simulink model "MX_Gateway"  (Simulink 9.0)
settings: Solver=FixedStepDiscrete  StartTime=0.0  StopTime=100  FixedStep=0.01  RelTol=1e-3
values from neighbouring files (symbols this model uses):
  ACTIVE = Bobcat.Parameter; CreateDefine( ACTIVE                         ,1                          ,'Define' ,'defines.h' )    [defines.m]
  ACTIVE_MODE_ONE = Bobcat.Parameter; CreateDefine( ACTIVE_MODE_ONE                ,19                         ,'Define' ,'defines.h' )    [defines.m]
  AUTHENTICATED = Bobcat.Parameter; CreateDefine( AUTHENTICATED                  ,1                          ,'Define' ,'defines.h' )    [defines.m]
  AUX_EMEA_CONTROLS = Bobcat.Parameter; CreateDefine( AUX_EMEA_CONTROLS  ,1   ,'Define' ,'defines.h' )    [enums.m]
  AUX_FLOW_HIGH = Bobcat.Parameter; CreateDefine( AUX_FLOW_HIGH           ,4  ,'Define' ,'defines.h' )    [enums.m]
  AUX_FLOW_INACTIVE = Bobcat.Parameter; CreateDefine( AUX_FLOW_INACTIVE       ,0  ,'Define' ,'defines.h' )    [enums.m]
  AUX_FLOW_LOW = Bobcat.Parameter; CreateDefine( AUX_FLOW_LOW            ,1  ,'Define' ,'defines.h' )    [enums.m]
  AUX_FLOW_MEDIUM = Bobcat.Parameter; CreateDefine( AUX_FLOW_MEDIUM         ,2  ,'Define' ,'defines.h' )    [enums.m]
  AUX_FLOW_STANDARD = Bobcat.Parameter; CreateDefine( AUX_FLOW_STANDARD       ,3  ,'Define' ,'defines.h' )    [enums.m]
  AUX_NON_FUNCTIONAL = Bobcat.Parameter; CreateDefine( AUX_NON_FUNCTIONAL ,255 ,'Define' ,'defines.h' )    [enums.m]
  BASE_ACTIVE = Bobcat.Parameter; CreateDefine( BASE_ACTIVE                    ,253                        ,'Define' ,'defines.h' )    [defines.m]
  BASE_DETENT = Bobcat.Parameter; CreateDefine( BASE_DETENT                    ,1                          ,'Define' ,'defines.h' )    [defines.m]
  BEEP_NONE = Bobcat.Parameter; CreateDefine( BEEP_NONE                      ,255                        ,'Define' ,'defines.h' )    [defines.m]
  CD_AIR_FILTER_DEBOUNCE_NORMAL = Bobcat.Parameter; CreateAliasCD( CD_AIR_FILTER_DEBOUNCE_NORMAL                        ,uint16(500)                ,'ConstantDataPointer' ,'CONSTANT_DATA' ,'AIR_FILTER_DEBOUNCE_NORMAL')    [cdata.m]    (also in hc12_cdata.m; m4_cdata.m)
  CD_AIR_FILTER_DEBOUNCE_NOT_CONNECTED = Bobcat.Parameter; CreateAliasCD( CD_AIR_FILTER_DEBOUNCE_NOT_CONNECTED                 ,uint16(3000)               ,'ConstantDataPointer' ,'CONSTANT_DATA' ,'AIR_FILTER_DEBOUNCE_NOT_CONNECTED')    [cdata.m]    (also in hc12_cdata.m; m4_cdata.m)
  CD_AIR_FILTER_DEBOUNCE_PLUGGED = Bobcat.Parameter; CreateAliasCD( CD_AIR_FILTER_DEBOUNCE_PLUGGED                       ,uint16(3000)               ,'ConstantDataPointer' ,'CONSTANT_DATA' ,'AIR_FILTER_DEBOUNCE_PLUGGED')    [cdata.m]    (also in hc12_cdata.m; m4_cdata.m)
  CD_AIR_FILTER_FUNCTION = Bobcat.Parameter; CreateAliasCD( CD_AIR_FILTER_FUNCTION                               ,uint8(255)                 ,'ConstantDataPointer' ,'CONSTANT_DATA' ,'AIR_FILTER_FUNCTION')    [cdata.m]    (also in hc12_cdata.m; m4_cdata.m)
  CD_AIR_FILTER_PLUGGED_WEIGHT = Bobcat.Parameter; CreateAliasCD( CD_AIR_FILTER_PLUGGED_WEIGHT                         ,uint8(2)                   ,'ConstantDataPointer' ,'CONSTANT_DATA' ,'AIR_FILTER_PLUGGED_WEIGHT')    [cdata.m]    (also in hc12_cdata.m; m4_cdata.m)
  CD_AIR_FILTER_UNPLUGGED_WEIGHT = Bobcat.Parameter; CreateAliasCD( CD_AIR_FILTER_UNPLUGGED_WEIGHT                       ,uint8(1)                   ,'ConstantDataPointer' ,'CONSTANT_DATA' ,'AIR_FILTER_UNPLUGGED_WEIGHT')    [cdata.m]    (also in hc12_cdata.m; m4_cdata.m)
  CD_AUTOIDLE_ARMBAR_DELAY = Bobcat.Parameter; CreateAliasCD( CD_AUTOIDLE_ARMBAR_DELAY                             ,uint8(2)                   ,'ConstantDataPointer' ,'CONSTANT_DATA' ,'AUTOIDLE_ARMBAR_DELAY')    [cdata.m]    (also in hc12_cdata.m; m4_cdata.m)
  CD_AUTOIDLE_FUNCTION = Bobcat.Parameter; CreateAliasCD( CD_AUTOIDLE_FUNCTION                                 ,uint8(255)                 ,'ConstantDataPointer' ,'CONSTANT_DATA' ,'AUTOIDLE_FUNCTION')    [cdata.m]    (also in hc12_cdata.m; m4_cdata.m)
  CD_AUTOIDLE_IDLE_DELAY = Bobcat.Parameter; CreateAliasCD( CD_AUTOIDLE_IDLE_DELAY                               ,uint8(40)                  ,'ConstantDataPointer' ,'CONSTANT_DATA' ,'AUTOIDLE_IDLE_DELAY')    [cdata.m]    (also in hc12_cdata.m; m4_cdata.m)
  CD_AUTOIDLE_LOW_IDLE_START = Bobcat.Parameter; CreateAliasCD( CD_AUTOIDLE_LOW_IDLE_START                           ,uint8(255)                 ,'ConstantDataPointer' ,'CONSTANT_DATA' ,'AUTOIDLE_LOW_IDLE_START')    [cdata.m]    (also in hc12_cdata.m; m4_cdata.m)
  CD_AUTOIDLE_PRESSURE_OFF = Bobcat.Parameter; CreateAliasCD( CD_AUTOIDLE_PRESSURE_OFF                             ,uint16(300)                ,'ConstantDataPointer' ,'CONSTANT_DATA' ,'AUTOIDLE_PRESSURE_OFF')    [cdata.m]    (also in hc12_cdata.m; m4_cdata.m)
  CD_AUTOIDLE_PRESSURE_ON = Bobcat.Parameter; CreateAliasCD( CD_AUTOIDLE_PRESSURE_ON                              ,uint16(270)                ,'ConstantDataPointer' ,'CONSTANT_DATA' ,'AUTOIDLE_PRESSURE_ON')    [cdata.m]    (also in hc12_cdata.m; m4_cdata.m)
  CD_AUTOSHIFT_FUNCTION = Bobcat.Parameter; CreateAliasCD( CD_AUTOSHIFT_FUNCTION                                ,uint8(255)                 ,'ConstantDataPointer' ,'CONSTANT_DATA' ,'AUTOSHIFT_FUNCTION')    [cdata.m]    (also in hc12_cdata.m; m4_cdata.m)
  CD_AUTOSHIFT_PHIGH1 = Bobcat.Parameter; CreateAliasCD( CD_AUTOSHIFT_PHIGH1                                  ,uint16(205)                ,'ConstantDataPointer' ,'CONSTANT_DATA' ,'AUTOSHIFT_PHIGH1')    [cdata.m]    (also in hc12_cdata.m; m4_cdata.m)
  CD_AUTOSHIFT_PHIGH2 = Bobcat.Parameter; CreateAliasCD( CD_AUTOSHIFT_PHIGH2                                  ,uint16(193)                ,'ConstantDataPointer' ,'CONSTANT_DATA' ,'AUTOSHIFT_PHIGH2')    [cdata.m]    (also in hc12_cdata.m; m4_cdata.m)
  CD_AUTOSHIFT_PHIGH_TEMP1 = Bobcat.Parameter; CreateAliasCD( CD_AUTOSHIFT_PHIGH_TEMP1                             ,int16(70)                  ,'ConstantDataPointer' ,'CONSTANT_DATA' ,'AUTOSHIFT_PHIGH_TEMP1')    [cdata.m]    (also in hc12_cdata.m; m4_cdata.m)
  CD_AUTOSHIFT_PHIGH_TEMP2 = Bobcat.Parameter; CreateAliasCD( CD_AUTOSHIFT_PHIGH_TEMP2                             ,int16(160)                 ,'ConstantDataPointer' ,'CONSTANT_DATA' ,'AUTOSHIFT_PHIGH_TEMP2')    [cdata.m]    (also in hc12_cdata.m; m4_cdata.m)
  CD_AUTOSHIFT_PLOW1 = Bobcat.Parameter; CreateAliasCD( CD_AUTOSHIFT_PLOW1                                   ,uint16(130)                ,'ConstantDataPointer' ,'CONSTANT_DATA' ,'AUTOSHIFT_PLOW1')    [cdata.m]    (also in hc12_cdata.m; m4_cdata.m)
  CD_AUTOSHIFT_PLOW2 = Bobcat.Parameter; CreateAliasCD( CD_AUTOSHIFT_PLOW2                                   ,uint16(100)                ,'ConstantDataPointer' ,'CONSTANT_DATA' ,'AUTOSHIFT_PLOW2')    [cdata.m]    (also in hc12_cdata.m; m4_cdata.m)
  CD_AUTOSHIFT_PLOW_TEMP1 = Bobcat.Parameter; CreateAliasCD( CD_AUTOSHIFT_PLOW_TEMP1                              ,int16(20)                  ,'ConstantDataPointer' ,'CONSTANT_DATA' ,'AUTOSHIFT_PLOW_TEMP1')    [cdata.m]    (also in hc12_cdata.m; m4_cdata.m)
  CD_AUTOSHIFT_PLOW_TEMP2 = Bobcat.Parameter; CreateAliasCD( CD_AUTOSHIFT_PLOW_TEMP2                              ,int16(80)                  ,'ConstantDataPointer' ,'CONSTANT_DATA' ,'AUTOSHIFT_PLOW_TEMP2')    [cdata.m]    (also in hc12_cdata.m; m4_cdata.m)
  CD_AUTOSHIFT_TRAVEL_DELAY = Bobcat.Parameter; CreateAliasCD( CD_AUTOSHIFT_TRAVEL_DELAY                            ,uint8(32)                  ,'ConstantDataPointer' ,'CONSTANT_DATA' ,'AUTOSHIFT_TRAVEL_DELAY')    [cdata.m]    (also in hc12_cdata.m; m4_cdata.m)
  CD_AUX2_CRACKPOINT_CURRENT = Bobcat.Parameter; CreateAliasCD( CD_AUX2_CRACKPOINT_CURRENT                           ,fi(0,0,16,12,'hex','0c28') ,'ConstantDataPointer' ,'CONSTANT_DATA' ,'AUX2_CRACKPOINT_CURRENT')    [cdata.m]    (also in hc12_cdata.m; m4_cdata.m)
  CD_AUX2_FLOW_LOW_FUNCTION = Bobcat.Parameter; CreateAliasCD( CD_AUX2_FLOW_LOW_FUNCTION                            ,uint8(255)                 ,'ConstantDataPointer' ,'CONSTANT_DATA' ,'AUX2_FLOW_LOW_FUNCTION')    [cdata.m]    (also in hc12_cdata.m; m4_cdata.m)
  CD_AUX2_FLOW_MED_FUNCTION = Bobcat.Parameter; CreateAliasCD( CD_AUX2_FLOW_MED_FUNCTION                            ,uint8(255)                 ,'ConstantDataPointer' ,'CONSTANT_DATA' ,'AUX2_FLOW_MED_FUNCTION')    [cdata.m]    (also in hc12_cdata.m; m4_cdata.m)
  CD_AUX2_HI_FLOW_PERCENT = Bobcat.Parameter; CreateAliasCD( CD_AUX2_HI_FLOW_PERCENT                              ,fi(0,0,16,16,'hex','ffff') ,'ConstantDataPointer' ,'CONSTANT_DATA' ,'AUX2_HI_FLOW_PERCENT')    [cdata.m]    (also in hc12_cdata.m; m4_cdata.m)
  CD_AUX2_LOW_FLOW_PERCENT = Bobcat.Parameter; CreateAliasCD( CD_AUX2_LOW_FLOW_PERCENT                             ,fi(0,0,16,16,'hex','7333') ,'ConstantDataPointer' ,'CONSTANT_DATA' ,'AUX2_LOW_FLOW_PERCENT')    [cdata.m]    (also in hc12_cdata.m; m4_cdata.m)
  CD_AUX2_MAX_FLOW_CURRENT = Bobcat.Parameter; CreateAliasCD( CD_AUX2_MAX_FLOW_CURRENT                             ,fi(0,0,16,12,'hex','14cc') ,'ConstantDataPointer' ,'CONSTANT_DATA' ,'AUX2_MAX_FLOW_CURRENT')    [cdata.m]    (also in hc12_cdata.m; m4_cdata.m)
  CD_AUX2_MED_FLOW_PERCENT = Bobcat.Parameter; CreateAliasCD( CD_AUX2_MED_FLOW_PERCENT                             ,fi(0,0,16,16,'hex','9999') ,'ConstantDataPointer' ,'CONSTANT_DATA' ,'AUX2_MED_FLOW_PERCENT')    [cdata.m]    (also in hc12_cdata.m; m4_cdata.m)
  CD_AUX_CRACKPOINT_CURRENT = Bobcat.Parameter; CreateAliasCD( CD_AUX_CRACKPOINT_CURRENT                            ,fi(0,0,16,12,'hex','0000') ,'ConstantDataPointer' ,'CONSTANT_DATA' ,'AUX_CRACKPOINT_CURRENT')    [cdata.m]    (also in hc12_cdata.m; m4_cdata.m)
  CD_AUX_DETENT_FLASH_TIME = Bobcat.Parameter; CreateAliasCD( CD_AUX_DETENT_FLASH_TIME                             ,uint16(72)                 ,'ConstantDataPointer' ,'CONSTANT_DATA' ,'AUX_DETENT_FLASH_TIME')    [cdata.m]    (also in hc12_cdata.m; m4_cdata.m)
  CD_AUX_DETENT_HOLD_TIME = Bobcat.Parameter; CreateAliasCD( CD_AUX_DETENT_HOLD_TIME                              ,uint16(100)                ,'ConstantDataPointer' ,'CONSTANT_DATA' ,'AUX_DETENT_HOLD_TIME')    [cdata.m]    (also in hc12_cdata.m; m4_cdata.m)
  CD_AUX_FULL_ON_AUX1 = Bobcat.Parameter; CreateAliasCD( CD_AUX_FULL_ON_AUX1                                  ,fi(0,0,16,12,'hex','10cd') ,'ConstantDataPointer' ,'CONSTANT_DATA' ,'AUX_FULL_ON_AUX1')    [cdata.m]    (also in hc12_cdata.m; m4_cdata.m)
  CD_AUX_FULL_ON_AUX2 = Bobcat.Parameter; CreateAliasCD( CD_AUX_FULL_ON_AUX2                                  ,fi(0,0,16,12,'hex','139d') ,'ConstantDataPointer' ,'CONSTANT_DATA' ,'AUX_FULL_ON_AUX2')    [cdata.m]    (also in hc12_cdata.m; m4_cdata.m)
  CD_AUX_FULL_ON_AUX3 = Bobcat.Parameter; CreateAliasCD( CD_AUX_FULL_ON_AUX3                                  ,fi(0,0,16,12,'hex','17d7') ,'ConstantDataPointer' ,'CONSTANT_DATA' ,'AUX_FULL_ON_AUX3')    [cdata.m]    (also in hc12_cdata.m; m4_cdata.m)
  CD_AUX_FUNCTION = Bobcat.Parameter; CreateAliasCD( CD_AUX_FUNCTION                                      ,uint8(0)                   ,'ConstantDataPointer' ,'CONSTANT_DATA' ,'AUX_FUNCTION')    [cdata.m]    (also in hc12_cdata.m; m4_cdata.m)
  CD_AUX_RATE_LIMIT_CRACKPOINT = Bobcat.Parameter; CreateAliasCD( CD_AUX_RATE_LIMIT_CRACKPOINT                         ,fi(0,0,16,12,'hex','0800') ,'ConstantDataPointer' ,'CONSTANT_DATA' ,'AUX_RATE_LIMIT_CRACKPOINT')    [cdata.m]    (also in hc12_cdata.m; m4_cdata.m)
  CD_AUX_RATE_LIMIT_CRACKPOINT_RATE = Bobcat.Parameter; CreateAliasCD( CD_AUX_RATE_LIMIT_CRACKPOINT_RATE                    ,fi(0,1,32,12,'int',102400) ,'ConstantDataPointer' ,'CONSTANT_DATA' ,'AUX_RATE_LIMIT_CRACKPOINT_RATE')    [cdata.m]    (also in hc12_cdata.m; m4_cdata.m)
  CD_AUX_RATE_LIMIT_FUNCTION = Bobcat.Parameter; CreateAliasCD( CD_AUX_RATE_LIMIT_FUNCTION                           ,uint8(255)                 ,'ConstantDataPointer' ,'CONSTANT_DATA' ,'AUX_RATE_LIMIT_FUNCTION')    [cdata.m]    (also in hc12_cdata.m; m4_cdata.m)
  CD_AUX_RATE_LIMIT_MAX_ENG_SPD = Bobcat.Parameter; CreateAliasCD( CD_AUX_RATE_LIMIT_MAX_ENG_SPD                        ,uint16(1600)               ,'ConstantDataPointer' ,'CONSTANT_DATA' ,'AUX_RATE_LIMIT_MAX_ENG_SPD')    [cdata.m]    (also in hc12_cdata.m; m4_cdata.m)
  CD_AUX_RATE_LIMIT_MIN_ENG_SPD = Bobcat.Parameter; CreateAliasCD( CD_AUX_RATE_LIMIT_MIN_ENG_SPD                        ,uint16(100)                ,'ConstantDataPointer' ,'CONSTANT_DATA' ,'AUX_RATE_LIMIT_MIN_ENG_SPD')    [cdata.m]    (also in hc12_cdata.m; m4_cdata.m)
  CD_AUX_RATE_LIMIT_NORMAL_RATE = Bobcat.Parameter; CreateAliasCD( CD_AUX_RATE_LIMIT_NORMAL_RATE                        ,fi(0,1,32,12,'int',2048)   ,'ConstantDataPointer' ,'CONSTANT_DATA' ,'AUX_RATE_LIMIT_NORMAL_RATE')    [cdata.m]    (also in hc12_cdata.m; m4_cdata.m)
  CD_AUXPOT_CAL_DEADBAND_HIGH = Bobcat.Parameter; CreateAliasCD( CD_AUXPOT_CAL_DEADBAND_HIGH                          ,uint16(574)                ,'ConstantDataPointer' ,'CONSTANT_DATA' ,'AUXPOT_CAL_DEADBAND_HIGH')    [cdata.m]    (also in hc12_cdata.m; m4_cdata.m)
  CD_AUXPOT_CAL_DEADBAND_LOW = Bobcat.Parameter; CreateAliasCD( CD_AUXPOT_CAL_DEADBAND_LOW                           ,uint16(424)                ,'ConstantDataPointer' ,'CONSTANT_DATA' ,'AUXPOT_CAL_DEADBAND_LOW')    [cdata.m]    (also in hc12_cdata.m; m4_cdata.m)
  CD_AUXPOT_CENTER_RATIO = Bobcat.Parameter; CreateAliasCD( CD_AUXPOT_CENTER_RATIO                               ,uint16(500)                ,'ConstantDataPointer' ,'CONSTANT_DATA' ,'AUXPOT_CENTER_RATIO')    [cdata.m]    (also in hc12_cdata.m; m4_cdata.m)
  CD_AUXPOT_DEADBAND = Bobcat.Parameter; CreateAliasCD( CD_AUXPOT_DEADBAND                                   ,uint8(38)                  ,'ConstantDataPointer' ,'CONSTANT_DATA' ,'AUXPOT_DEADBAND')    [cdata.m]    (also in hc12_cdata.m; m4_cdata.m)
  CD_AUXPOT_FUNCTION = Bobcat.Parameter; CreateAliasCD( CD_AUXPOT_FUNCTION                                   ,uint8(0)                   ,'ConstantDataPointer' ,'CONSTANT_DATA' ,'AUXPOT_FUNCTION')    [cdata.m]    (also in hc12_cdata.m; m4_cdata.m)
  CD_AUXPOT_RATIO_GAIN = Bobcat.Parameter; CreateAliasCD( CD_AUXPOT_RATIO_GAIN                                 ,uint16(1000)               ,'ConstantDataPointer' ,'CONSTANT_DATA' ,'AUXPOT_RATIO_GAIN')    [cdata.m]    (also in hc12_cdata.m; m4_cdata.m)
  CD_CAN_THROTTLE_FUNCTION = Bobcat.Parameter; CreateAliasCD( CD_CAN_THROTTLE_FUNCTION                             ,uint8(255)                 ,'ConstantDataPointer' ,'CONSTANT_DATA' ,'CAN_THROTTLE_FUNCTION')    [cdata.m]    (also in hc12_cdata.m; m4_cdata.m)
  CD_CAN_THROTTLE_REVERSE = Bobcat.Parameter; CreateAliasCD( CD_CAN_THROTTLE_REVERSE                              ,uint8(255)                 ,'ConstantDataPointer' ,'CONSTANT_DATA' ,'CAN_THROTTLE_REVERSE')    [cdata.m]    (also in hc12_cdata.m; m4_cdata.m)
  CD_CONSOLE_DEBOUNCE_ABSENT = Bobcat.Parameter; CreateAliasCD( CD_CONSOLE_DEBOUNCE_ABSENT                           ,uint16(12)                 ,'ConstantDataPointer' ,'CONSTANT_DATA' ,'CONSOLE_DEBOUNCE_ABSENT')    [cdata.m]    (also in hc12_cdata.m; m4_cdata.m)
  CD_CONSOLE_DEBOUNCE_OORH = Bobcat.Parameter; CreateAliasCD( CD_CONSOLE_DEBOUNCE_OORH                             ,uint16(12)                 ,'ConstantDataPointer' ,'CONSTANT_DATA' ,'CONSOLE_DEBOUNCE_OORH')    [cdata.m]    (also in hc12_cdata.m; m4_cdata.m)
  CD_CONSOLE_DEBOUNCE_OORL = Bobcat.Parameter; CreateAliasCD( CD_CONSOLE_DEBOUNCE_OORL                             ,uint16(12)                 ,'ConstantDataPointer' ,'CONSTANT_DATA' ,'CONSOLE_DEBOUNCE_OORL')    [cdata.m]    (also in hc12_cdata.m; m4_cdata.m)
  CD_CONSOLE_DEBOUNCE_PRESENT = Bobcat.Parameter; CreateAliasCD( CD_CONSOLE_DEBOUNCE_PRESENT                          ,uint16(12)                 ,'ConstantDataPointer' ,'CONSTANT_DATA' ,'CONSOLE_DEBOUNCE_PRESENT')    [cdata.m]    (also in hc12_cdata.m; m4_cdata.m)
  CD_CONSOLE_FUNCTION = Bobcat.Parameter; CreateAliasCD( CD_CONSOLE_FUNCTION                                  ,uint8(0)                   ,'ConstantDataPointer' ,'CONSTANT_DATA' ,'CONSOLE_FUNCTION')    [cdata.m]    (also in hc12_cdata.m; m4_cdata.m)
  CD_CONSOLE_OORH = Bobcat.Parameter; CreateAliasCD( CD_CONSOLE_OORH                                      ,uint16(740)                ,'ConstantDataPointer' ,'CONSTANT_DATA' ,'CONSOLE_OORH')    [cdata.m]    (also in hc12_cdata.m; m4_cdata.m)
  CD_CONSOLE_OORL = Bobcat.Parameter; CreateAliasCD( CD_CONSOLE_OORL                                      ,uint16(10)                 ,'ConstantDataPointer' ,'CONSTANT_DATA' ,'CONSOLE_OORL')    [cdata.m]    (also in hc12_cdata.m; m4_cdata.m)
  CD_CONSOLE_PRESENT_HIGH = Bobcat.Parameter; CreateAliasCD( CD_CONSOLE_PRESENT_HIGH                              ,uint16(515)                ,'ConstantDataPointer' ,'CONSTANT_DATA' ,'CONSOLE_PRESENT_HIGH')    [cdata.m]    (also in hc12_cdata.m; m4_cdata.m)
  CD_CONSOLE_PRESENT_LOW = Bobcat.Parameter; CreateAliasCD( CD_CONSOLE_PRESENT_LOW                               ,uint16(375)                ,'ConstantDataPointer' ,'CONSTANT_DATA' ,'CONSOLE_PRESENT_LOW')    [cdata.m]    (also in hc12_cdata.m; m4_cdata.m)
  CD_DELUXE_GEN5_5_FUNCTION = Bobcat.Parameter; CreateAliasCD( CD_DELUXE_GEN5_5_FUNCTION                            ,uint8(255)                 ,'ConstantDataPointer' ,'CONSTANT_DATA' ,'DELUXE_GEN5_5_FUNCTION')    [cdata.m]    (also in hc12_cdata.m; m4_cdata.m)
  CD_DELUXE_GEN5_FUNCTION = Bobcat.Parameter; CreateAliasCD( CD_DELUXE_GEN5_FUNCTION                              ,uint8(255)                 ,'ConstantDataPointer' ,'CONSTANT_DATA' ,'DELUXE_GEN5_FUNCTION')    [cdata.m]    (also in hc12_cdata.m; m4_cdata.m)
  CD_DERATE_ENGINE_SPEED_DEBOUNCE_HIGH = Bobcat.Parameter; CreateAliasCD( CD_DERATE_ENGINE_SPEED_DEBOUNCE_HIGH                 ,uint16(200)                ,'ConstantDataPointer' ,'CONSTANT_DATA' ,'DERATE_ENGINE_SPEED_DEBOUNCE_HIGH')    [cdata.m]    (also in hc12_cdata.m; m4_cdata.m)
  CD_DERATE_ENGINE_SPEED_DEBOUNCE_SHUTDOWN = Bobcat.Parameter; CreateAliasCD( CD_DERATE_ENGINE_SPEED_DEBOUNCE_SHUTDOWN             ,uint16(1000)               ,'ConstantDataPointer' ,'CONSTANT_DATA' ,'DERATE_ENGINE_SPEED_DEBOUNCE_SHUTDOWN')    [cdata.m]    (also in hc12_cdata.m; m4_cdata.m)
  CD_DERATE_ENGINE_SPEED_HIGH_OFFSET = Bobcat.Parameter; CreateAliasCD( CD_DERATE_ENGINE_SPEED_HIGH_OFFSET                   ,uint16(200)                ,'ConstantDataPointer' ,'CONSTANT_DATA' ,'DERATE_ENGINE_SPEED_HIGH_OFFSET')    [cdata.m]    (also in hc12_cdata.m; m4_cdata.m)
  CD_DERATE_METHOD = Bobcat.Parameter; CreateAliasCD( CD_DERATE_METHOD                                     ,uint8(0)                   ,'ConstantDataPointer' ,'CONSTANT_DATA' ,'DERATE_METHOD')    [cdata.m]    (also in hc12_cdata.m; m4_cdata.m)
  CD_DUMP_VALVE_FUNCTION = Bobcat.Parameter; CreateAliasCD( CD_DUMP_VALVE_FUNCTION                               ,uint8(255)                 ,'ConstantDataPointer' ,'CONSTANT_DATA' ,'DUMP_VALVE_FUNCTION')    [cdata.m]    (also in hc12_cdata.m; m4_cdata.m)
  CD_DUMP_VALVE_ON_CURRENT = Bobcat.Parameter; CreateAliasCD( CD_DUMP_VALVE_ON_CURRENT                             ,fi(0,0,16,12,'hex','ffff') ,'ConstantDataPointer' ,'CONSTANT_DATA' ,'DUMP_VALVE_ON_CURRENT')    [cdata.m]    (also in hc12_cdata.m; m4_cdata.m)
  CD_ECO_MODE_THROTTLE_REDUCTION = Bobcat.Parameter; CreateAliasCD( CD_ECO_MODE_THROTTLE_REDUCTION                       ,uint16(850)                ,'ConstantDataPointer' ,'CONSTANT_DATA' ,'ECO_MODE_THROTTLE_REDUCTION')    [cdata.m]    (also in hc12_cdata.m; m4_cdata.m)
  CD_ECT_FILTER_GAIN = Bobcat.Parameter; CreateAliasCD( CD_ECT_FILTER_GAIN                                   ,uint8(6)                   ,'ConstantDataPointer' ,'CONSTANT_DATA' ,'ECT_FILTER_GAIN')    [cdata.m]    (also in hc12_cdata.m; m4_cdata.m)
  CD_ECU_FUNCTION = Bobcat.Parameter; CreateAliasCD( CD_ECU_FUNCTION                                      ,uint8(255)                 ,'ConstantDataPointer' ,'CONSTANT_DATA' ,'ECU_FUNCTION')    [cdata.m]    (also in hc12_cdata.m; m4_cdata.m)
  CD_ECU_HOLD_DEBOUNCE_ERROR_ON = Bobcat.Parameter; CreateAliasCD( CD_ECU_HOLD_DEBOUNCE_ERROR_ON                        ,uint16(20)                 ,'ConstantDataPointer' ,'CONSTANT_DATA' ,'ECU_HOLD_DEBOUNCE_ERROR_ON')    [cdata.m]    (also in hc12_cdata.m; m4_cdata.m)
  CD_EMEA_CONTROLS_HOLD_TIME = Bobcat.Parameter; CreateAliasCD( CD_EMEA_CONTROLS_HOLD_TIME                           ,uint16(30)                 ,'ConstantDataPointer' ,'CONSTANT_DATA' ,'EMEA_CONTROLS_HOLD_TIME')    [cdata.m]    (also in hc12_cdata.m; m4_cdata.m)
  CD_EMEA_CONTROLS_OPTION_A = Bobcat.Parameter; CreateAliasCD( CD_EMEA_CONTROLS_OPTION_A                            ,uint8(255)                 ,'ConstantDataPointer' ,'CONSTANT_DATA' ,'EMEA_CONTROLS_OPTION_A')    [cdata.m]    (also in hc12_cdata.m; m4_cdata.m)
  CD_EMEA_CONTROLS_OPTION_C = Bobcat.Parameter; CreateAliasCD( CD_EMEA_CONTROLS_OPTION_C                            ,uint8(255)                 ,'ConstantDataPointer' ,'CONSTANT_DATA' ,'EMEA_CONTROLS_OPTION_C')    [cdata.m]    (also in hc12_cdata.m; m4_cdata.m)
  CD_EMEA_CONTROLS_REVERSE = Bobcat.Parameter; CreateAliasCD( CD_EMEA_CONTROLS_REVERSE                             ,uint8(255)                 ,'ConstantDataPointer' ,'CONSTANT_DATA' ,'EMEA_CONTROLS_REVERSE')    [cdata.m]    (also in hc12_cdata.m; m4_cdata.m)
  CD_EMEA_ELECTRIC_VERSION = Bobcat.Parameter; CreateAliasCD( CD_EMEA_ELECTRIC_VERSION                             ,uint8(0)                   ,'ConstantDataPointer' ,'CONSTANT_DATA' ,'EMEA_ELECTRIC_VERSION')    [cdata.m]    (also in hc12_cdata.m; m4_cdata.m)
  CD_EMEA_POT_CAL_DEADBAND_HIGH = Bobcat.Parameter; CreateAliasCD( CD_EMEA_POT_CAL_DEADBAND_HIGH                        ,uint16(574)                ,'ConstantDataPointer' ,'CONSTANT_DATA' ,'EMEA_POT_CAL_DEADBAND_HIGH')    [cdata.m]    (also in hc12_cdata.m; m4_cdata.m)
  CD_EMEA_POT_CAL_DEADBAND_LOW = Bobcat.Parameter; CreateAliasCD( CD_EMEA_POT_CAL_DEADBAND_LOW                         ,uint16(424)                ,'ConstantDataPointer' ,'CONSTANT_DATA' ,'EMEA_POT_CAL_DEADBAND_LOW')    [cdata.m]    (also in hc12_cdata.m; m4_cdata.m)
  CD_ENGINE_RPM_FACTOR = Bobcat.Parameter; CreateAliasCD( CD_ENGINE_RPM_FACTOR                                 ,uint8(35)                  ,'ConstantDataPointer' ,'CONSTANT_DATA' ,'ENGINE_RPM_FACTOR')    [cdata.m]    (also in hc12_cdata.m; m4_cdata.m)
  CD_ENGINE_SPEED_FILTER_FAST_VALUE = Bobcat.Parameter; CreateAliasCD( CD_ENGINE_SPEED_FILTER_FAST_VALUE                    ,uint8(1)                   ,'ConstantDataPointer' ,'CONSTANT_DATA' ,'ENGINE_SPEED_FILTER_FAST_VALUE')    [cdata.m]    (also in hc12_cdata.m; m4_cdata.m)
  CD_ENGINE_SPEED_FILTER_SLOW_VALUE = Bobcat.Parameter; CreateAliasCD( CD_ENGINE_SPEED_FILTER_SLOW_VALUE                    ,uint8(4)                   ,'ConstantDataPointer' ,'CONSTANT_DATA' ,'ENGINE_SPEED_FILTER_SLOW_VALUE')    [cdata.m]    (also in hc12_cdata.m; m4_cdata.m)
  CD_ENGINE_SPEED_RUNNING = Bobcat.Parameter; CreateAliasCD( CD_ENGINE_SPEED_RUNNING                              ,uint16(800)                ,'ConstantDataPointer' ,'CONSTANT_DATA' ,'ENGINE_SPEED_RUNNING')    [cdata.m]    (also in hc12_cdata.m; m4_cdata.m)
  CD_ENGINE_START_DELAY_DEBOUNCE_RPM_NO_SIGNAL = Bobcat.Parameter; CreateAliasCD( CD_ENGINE_START_DELAY_DEBOUNCE_RPM_NO_SIGNAL         ,uint16(0)                  ,'ConstantDataPointer' ,'CONSTANT_DATA' ,'ENGINE_START_DELAY_DEBOUNCE_RPM_NO_SIGNAL')    [cdata.m]    (also in hc12_cdata.m; m4_cdata.m)
  CD_ENGINE_START_DELAY_OFF_TIME = Bobcat.Parameter; CreateAliasCD( CD_ENGINE_START_DELAY_OFF_TIME                       ,uint16(0)                  ,'ConstantDataPointer' ,'CONSTANT_DATA' ,'ENGINE_START_DELAY_OFF_TIME')    [cdata.m]    (also in hc12_cdata.m; m4_cdata.m)
  CD_ENGINE_START_DELAY_RPM_NO_SIGNAL = Bobcat.Parameter; CreateAliasCD( CD_ENGINE_START_DELAY_RPM_NO_SIGNAL                  ,uint16(0)                  ,'ConstantDataPointer' ,'CONSTANT_DATA' ,'ENGINE_START_DELAY_RPM_NO_SIGNAL')    [cdata.m]    (also in hc12_cdata.m; m4_cdata.m)
  CD_EOP_DEBOUNCE_EXTREMELY_LOW = Bobcat.Parameter; CreateAliasCD( CD_EOP_DEBOUNCE_EXTREMELY_LOW                        ,uint16(50)                 ,'ConstantDataPointer' ,'CONSTANT_DATA' ,'EOP_DEBOUNCE_EXTREMELY_LOW')    [cdata.m]    (also in hc12_cdata.m; m4_cdata.m)
  CD_EOP_DEBOUNCE_NORMAL = Bobcat.Parameter; CreateAliasCD( CD_EOP_DEBOUNCE_NORMAL                               ,uint16(50)                 ,'ConstantDataPointer' ,'CONSTANT_DATA' ,'EOP_DEBOUNCE_NORMAL')    [cdata.m]    (also in hc12_cdata.m; m4_cdata.m)
  CD_EOP_DEBOUNCE_SHUTDOWN = Bobcat.Parameter; CreateAliasCD( CD_EOP_DEBOUNCE_SHUTDOWN                             ,uint16(100)                ,'ConstantDataPointer' ,'CONSTANT_DATA' ,'EOP_DEBOUNCE_SHUTDOWN')    [cdata.m]    (also in hc12_cdata.m; m4_cdata.m)
  CD_EOP_FILTER_VALUE = Bobcat.Parameter; CreateAliasCD( CD_EOP_FILTER_VALUE                                  ,uint8(3)                   ,'ConstantDataPointer' ,'CONSTANT_DATA' ,'EOP_FILTER_VALUE')    [cdata.m]    (also in hc12_cdata.m; m4_cdata.m)
  CD_EOP_FUNCTION = Bobcat.Parameter; CreateAliasCD( CD_EOP_FUNCTION                                      ,uint8(1)                   ,'ConstantDataPointer' ,'CONSTANT_DATA' ,'EOP_FUNCTION')    [cdata.m]    (also in hc12_cdata.m; m4_cdata.m)
  CD_EOP_SWITCH_GAUGE_VALUE = Bobcat.Parameter; CreateAliasCD( CD_EOP_SWITCH_GAUGE_VALUE                            ,uint16(125)                ,'ConstantDataPointer' ,'CONSTANT_DATA' ,'EOP_SWITCH_GAUGE_VALUE')    [cdata.m]    (also in hc12_cdata.m; m4_cdata.m)
  CD_EOP_SWITCH_LOW = Bobcat.Parameter; CreateAliasCD( CD_EOP_SWITCH_LOW                                    ,uint16(50)                 ,'ConstantDataPointer' ,'CONSTANT_DATA' ,'EOP_SWITCH_LOW')    [cdata.m]    (also in hc12_cdata.m; m4_cdata.m)
  CD_EOP_SWITCH_PRESSURE_VALUE = Bobcat.Parameter; CreateAliasCD( CD_EOP_SWITCH_PRESSURE_VALUE                         ,uint16(40)                 ,'ConstantDataPointer' ,'CONSTANT_DATA' ,'EOP_SWITCH_PRESSURE_VALUE')    [cdata.m]    (also in hc12_cdata.m; m4_cdata.m)
  CD_EOT_DEBOUNCE_EXTREMELY_LOW = Bobcat.Parameter; CreateAliasCD( CD_EOT_DEBOUNCE_EXTREMELY_LOW                        ,uint16(2)                  ,'ConstantDataPointer' ,'CONSTANT_DATA' ,'EOT_DEBOUNCE_EXTREMELY_LOW')    [cdata.m]    (also in hc12_cdata.m; m4_cdata.m)
  CD_EOT_DEBOUNCE_LOW = Bobcat.Parameter; CreateAliasCD( CD_EOT_DEBOUNCE_LOW                                  ,uint16(2)                  ,'ConstantDataPointer' ,'CONSTANT_DATA' ,'EOT_DEBOUNCE_LOW')    [cdata.m]    (also in hc12_cdata.m; m4_cdata.m)
  CD_EOT_DEBOUNCE_NORMAL = Bobcat.Parameter; CreateAliasCD( CD_EOT_DEBOUNCE_NORMAL                               ,uint16(2)                  ,'ConstantDataPointer' ,'CONSTANT_DATA' ,'EOT_DEBOUNCE_NORMAL')    [cdata.m]    (also in hc12_cdata.m; m4_cdata.m)
  CD_EOT_EXTREMELY_LOW = Bobcat.Parameter; CreateAliasCD( CD_EOT_EXTREMELY_LOW                                 ,uint16(81)                 ,'ConstantDataPointer' ,'CONSTANT_DATA' ,'EOT_EXTREMELY_LOW')    [cdata.m]    (also in hc12_cdata.m; m4_cdata.m)
  CD_EOT_FUNCTION = Bobcat.Parameter; CreateAliasCD( CD_EOT_FUNCTION                                      ,uint8(255)                 ,'ConstantDataPointer' ,'CONSTANT_DATA' ,'EOT_FUNCTION')    [cdata.m]    (also in hc12_cdata.m; m4_cdata.m)
  CD_EOT_LOW = Bobcat.Parameter; CreateAliasCD( CD_EOT_LOW                                           ,uint16(90)                 ,'ConstantDataPointer' ,'CONSTANT_DATA' ,'EOT_LOW')    [cdata.m]    (also in hc12_cdata.m; m4_cdata.m)
  CD_EOT_TEMPERATURE_HYSTERESIS = Bobcat.Parameter; CreateAliasCD( CD_EOT_TEMPERATURE_HYSTERESIS                        ,uint16(2)                  ,'ConstantDataPointer' ,'CONSTANT_DATA' ,'EOT_TEMPERATURE_HYSTERESIS')    [cdata.m]    (also in hc12_cdata.m; m4_cdata.m)
  CD_FAST_TRAVEL_FUNCTION = Bobcat.Parameter; CreateAliasCD( CD_FAST_TRAVEL_FUNCTION                              ,uint8(255)                 ,'ConstantDataPointer' ,'CONSTANT_DATA' ,'FAST_TRAVEL_FUNCTION')    [cdata.m]    (also in hc12_cdata.m; m4_cdata.m)
  CD_FAST_TRAVEL_THROTTLE_DELAY = Bobcat.Parameter; CreateAliasCD( CD_FAST_TRAVEL_THROTTLE_DELAY                        ,uint16(10)                 ,'ConstantDataPointer' ,'CONSTANT_DATA' ,'FAST_TRAVEL_THROTTLE_DELAY')    [cdata.m]    (also in hc12_cdata.m; m4_cdata.m)
  CD_FAST_TRAVEL_THROTTLE_LIMIT = Bobcat.Parameter; CreateAliasCD( CD_FAST_TRAVEL_THROTTLE_LIMIT                        ,uint16(1000)               ,'ConstantDataPointer' ,'CONSTANT_DATA' ,'FAST_TRAVEL_THROTTLE_LIMIT')    [cdata.m]    (also in hc12_cdata.m; m4_cdata.m)
  CD_FLYWHEEL_TEETH = Bobcat.Parameter; CreateAliasCD( CD_FLYWHEEL_TEETH                                    ,uint8(83)                  ,'ConstantDataPointer' ,'CONSTANT_DATA' ,'FLYWHEEL_TEETH')    [cdata.m]    (also in hc12_cdata.m; m4_cdata.m)
  CD_FUEL_LEVEL_FILTER_FAST_VALUE = Bobcat.Parameter; CreateAliasCD( CD_FUEL_LEVEL_FILTER_FAST_VALUE                      ,uint8(5)                   ,'ConstantDataPointer' ,'CONSTANT_DATA' ,'FUEL_LEVEL_FILTER_FAST_VALUE')    [cdata.m]    (also in hc12_cdata.m; m4_cdata.m)
  CD_FUEL_LEVEL_FILTER_SLOW_VALUE = Bobcat.Parameter; CreateAliasCD( CD_FUEL_LEVEL_FILTER_SLOW_VALUE                      ,uint8(10)                  ,'ConstantDataPointer' ,'CONSTANT_DATA' ,'FUEL_LEVEL_FILTER_SLOW_VALUE')    [cdata.m]    (also in hc12_cdata.m; m4_cdata.m)
  CD_FUEL_PRIMARY_FEEDBACK_FUNCTION = Bobcat.Parameter; CreateAliasCD( CD_FUEL_PRIMARY_FEEDBACK_FUNCTION                    ,uint8(0)                   ,'ConstantDataPointer' ,'CONSTANT_DATA' ,'FUEL_PRIMARY_FEEDBACK_FUNCTION')    [cdata.m]    (also in hc12_cdata.m; m4_cdata.m)
  ... (+246 more)
undefined symbols (not defined in the file or in its folder): sigbuilder_block
undefined symbols (not defined in the file): CD_FUEL_RESOLUTION_TIMER
libraries: simulink x186, MX_Library x107
(part 27 of 48)

== stateflow chart MX_Gtwy_Control/Inputs/VBattery/VBattery_State ==
  state NON_FUNCTIONAL
    en:state_VBattery = NORMAL;
    en:state_Alternator = NORMAL;
  state NORMAL
    state EXTREMELY_HIGH
      state DEBOUNCE
        en:debounceCount = 0;
        du:debounceCount++;
      state EXTREMELY_HIGH
        en:state_VBattery = EXTREMELY_HIGH;
        en:state_Alternator = NORMAL;
      (default) -> NORMAL.EXTREMELY_HIGH.DEBOUNCE
      NORMAL.EXTREMELY_HIGH.DEBOUNCE -> NORMAL.EXTREMELY_HIGH.EXTREMELY_HIGH : [(debounceCount >= CD_VBATTERY_DEBOUNCE_EXTREMELY_HIGH)...
          | || (state_VBattery == EXTREMELY_HIGH)]
    state HIGH
      state DEBOUNCE
        en:debounceCount = 0;
        du:debounceCount++;
      state HIGH
        en:state_VBattery = HIGH;
        en:state_Alternator = NORMAL;
      (default) -> NORMAL.HIGH.DEBOUNCE
      NORMAL.HIGH.DEBOUNCE -> NORMAL.HIGH.HIGH : [(debounceCount >= CD_VBATTERY_DEBOUNCE_HIGH)...
          | || (state_VBattery == HIGH)]
    state ALTERNATOR_HIGH
      state DEBOUNCE
        en:debounceCount = 0;
        du:debounceCount++;
      state HIGH
        en:state_Alternator = HIGH;
        en:state_VBattery = NORMAL;
      (default) -> NORMAL.ALTERNATOR_HIGH.DEBOUNCE
      NORMAL.ALTERNATOR_HIGH.DEBOUNCE -> NORMAL.ALTERNATOR_HIGH.HIGH : [(debounceCount >= CD_ALTERNATOR_DEBOUNCE_HIGH)]
    state NORMAL
      state DEBOUNCE
        en:debounceCount = 0;
        du:debounceCount++;
      state NORMAL
        en:state_VBattery = NORMAL;
        en:state_Alternator = NORMAL;
      (default) -> NORMAL.NORMAL.DEBOUNCE
      NORMAL.NORMAL.DEBOUNCE -> NORMAL.NORMAL.NORMAL : [(debounceCount >= CD_VBATTERY_DEBOUNCE_NORMAL)...
          | || ((state_VBattery == NORMAL) && (state_Alternator == NORMAL))]
    state LOW
      state DEBOUNCE
        en:debounceCount = 0;
        du:debounceCount++;
      state LOW
        en:state_VBattery = LOW;
        en:state_Alternator = NORMAL;
      (default) -> NORMAL.LOW.DEBOUNCE
      NORMAL.LOW.DEBOUNCE -> NORMAL.LOW.LOW : [(debounceCount >= CD_VBATTERY_DEBOUNCE_LOW)...
          | || (state_VBattery == LOW)]
    state ALTERNATOR_LOW
      state DEBOUNCE
        en:debounceCount = 0;
        du:debounceCount++;
      state LOW
        en:state_Alternator = LOW;
        en:state_VBattery = NORMAL;
      (default) -> NORMAL.ALTERNATOR_LOW.DEBOUNCE
      NORMAL.ALTERNATOR_LOW.DEBOUNCE -> NORMAL.ALTERNATOR_LOW.LOW : [(debounceCount >= CD_ALTERNATOR_DEBOUNCE_LOW)]
    state EXTREMELY_LOW
      state DEBOUNCE
        en:debounceCount = 0;
        du:debounceCount++;
      state EXTREMELY_LOW
        en:state_VBattery = EXTREMELY_LOW;
        en:state_Alternator = NORMAL;
      (default) -> NORMAL.EXTREMELY_LOW.DEBOUNCE
      NORMAL.EXTREMELY_LOW.DEBOUNCE -> NORMAL.EXTREMELY_LOW.EXTREMELY_LOW : [(debounceCount >= CD_VBATTERY_DEBOUNCE_EXTREMELY_LOW)...
          | || (state_VBattery == EXTREMELY_LOW)]
    ALTERNATOR_HIGH -> NORMAL.EXTREMELY_HIGH [order 2] : [(calc_VBattery >= CD_VBATTERY_EXTREMELY_HIGH)]
    NORMAL.EXTREMELY_HIGH -> ALTERNATOR_HIGH [order 1] : [(calc_VBattery < (CD_VBATTERY_EXTREMELY_HIGH - CD_VOLTAGE_HYSTERESIS))...
        | && ((CD_ALTERNATOR_FUNCTION != NON_FUNCTIONAL) && (flt_EngineSpeed >= CD_ALTERNATOR_ALARM_ENGINE_SPEED))]
    NORMAL.HIGH -> NORMAL.EXTREMELY_HIGH [order 1] : [(calc_VBattery >= CD_VBATTERY_EXTREMELY_HIGH)]
    NORMAL.EXTREMELY_HIGH -> NORMAL.HIGH [order 2] : [(calc_VBattery < (CD_VBATTERY_EXTREMELY_HIGH - CD_VOLTAGE_HYSTERESIS))...
        | && ((CD_ALTERNATOR_FUNCTION ==NON_FUNCTIONAL)...
        |   || (flt_EngineSpeed < CD_ALTERNATOR_ALARM_ENGINE_SPEED))]
    NORMAL.NORMAL -> NORMAL.HIGH [order 3] : [(calc_VBattery >= CD_VBATTERY_HIGH)...
        | && ((CD_ALTERNATOR_FUNCTION ==NON_FUNCTIONAL)...
        |    ||  (flt_EngineSpeed < CD_ALTERNATOR_ALARM_ENGINE_SPEED))]
    NORMAL.HIGH -> NORMAL.NORMAL [order 2] : [(calc_VBattery < (CD_VBATTERY_HIGH - CD_VOLTAGE_HYSTERESIS))]
    ALTERNATOR_HIGH -> NORMAL.NORMAL [order 1] : [(calc_VBattery < (CD_ALTERNATOR_HIGH - CD_VOLTAGE_HYSTERESIS))...
        | || (flt_EngineSpeed < CD_ALTERNATOR_ALARM_ENGINE_SPEED)]
    NORMAL.NORMAL -> ALTERNATOR_HIGH [order 2] : [(CD_ALTERNATOR_FUNCTION != NON_FUNCTIONAL)...
        | && (calc_VBattery >= CD_ALTERNATOR_HIGH)...
        | && (flt_EngineSpeed >= CD_ALTERNATOR_ALARM_ENGINE_SPEED)]
    (default) -> NORMAL.NORMAL
    NORMAL.NORMAL -> ALTERNATOR_LOW [order 1] : [(CD_ALTERNATOR_FUNCTION != NON_FUNCTIONAL)...
        | && (calc_VBattery <= CD_ALTERNATOR_LOW)...
        | && (flt_EngineSpeed >= CD_ALTERNATOR_ALARM_ENGINE_SPEED)]
    ALTERNATOR_LOW -> NORMAL.NORMAL [order 1] : [(calc_VBattery > (CD_ALTERNATOR_LOW + CD_VOLTAGE_HYSTERESIS))...
        | || (flt_EngineSpeed < CD_ALTERNATOR_ALARM_ENGINE_SPEED)]
    NORMAL.NORMAL -> NORMAL.LOW [order 4] : [(calc_VBattery <= CD_VBATTERY_LOW)...
        | && ((CD_ALTERNATOR_FUNCTION == NON_FUNCTIONAL)...
        |   ||  (flt_EngineSpeed < CD_ALTERNATOR_ALARM_ENGINE_SPEED))]
    NORMAL.LOW -> NORMAL.NORMAL [order 1] : [(calc_VBattery > (CD_VBATTERY_LOW + CD_VOLTAGE_HYSTERESIS))...
        | && (state_Engine != ENGINE_CRANKING)]
    NORMAL.LOW -> NORMAL.EXTREMELY_LOW [order 2] : [(calc_VBattery <= CD_VBATTERY_EXTREMELY_LOW)]
    NORMAL.EXTREMELY_LOW -> NORMAL.LOW [order 2] : [(calc_VBattery > (CD_VBATTERY_EXTREMELY_LOW + CD_VOLTAGE_HYSTERESIS))...
        | && (state_Engine != ENGINE_CRANKING)...
        | && ((CD_ALTERNATOR_FUNCTION == NON_FUNCTIONAL)...
        |   ||  (flt_EngineSpeed < CD_ALTERNATOR_ALARM_ENGINE_SPEED))]
    NORMAL.EXTREMELY_LOW -> ALTERNATOR_LOW [order 1] : [(calc_VBattery > (CD_VBATTERY_EXTREMELY_LOW + CD_VOLTAGE_HYSTERESIS))...
        | && ((CD_ALTERNATOR_FUNCTION != NON_FUNCTIONAL) && (flt_EngineSpeed >= CD_ALTERNATOR_ALARM_ENGINE_SPEED))]
    ALTERNATOR_LOW -> NORMAL.EXTREMELY_LOW [order 2] : [(calc_VBattery <= CD_VBATTERY_EXTREMELY_LOW)]
  state OORL
    state DEBOUNCE_OORL
      en:debounceCount = 0;
      du:debounceCount++;
    state OORL
      en:state_VBattery = OORL;
      en:state_Alternator = NORMAL;
    (default) -> DEBOUNCE_OORL
    DEBOUNCE_OORL -> OORL.OORL : [(debounceCount >= CD_VBATTERY_DEBOUNCE_OORL)...
        | || (state_VBattery == OORL)]
  (default) -> NON_FUNCTIONAL
  NON_FUNCTIONAL -> NORMAL : [(CD_VBATTERY_FUNCTION != NON_FUNCTIONAL)]
  NORMAL -> OORL : [(calc_VBattery <= CD_VBATTERY_OORL)]
  OORL -> NORMAL : [(state_Engine != ENGINE_CRANKING)...
      | && (calc_VBattery > (CD_VBATTERY_OORL + CD_VOLTAGE_HYSTERESIS))]
  data: state_VBattery output uint8; calc_VBattery input fixdt(0,16,8); debounceCount local uint16 =0; CD_VBATTERY_DEBOUNCE_EXTREMELY_LOW parameter uint16; CD_VBATTERY_DEBOUNCE_HIGH parameter uint16; CD_VBATTERY_DEBOUNCE_LOW parameter uint16; CD_VBATTERY_DEBOUNCE_NORMAL parameter uint16; CD_VBATTERY_DEBOUNCE_OORL parameter uint16; CD_VBATTERY_EXTREMELY_LOW parameter uint16; CD_VBATTERY_FUNCTION parameter uint8; CD_VBATTERY_HIGH parameter uint8; CD_VBATTERY_LOW parameter uint8; CD_VBATTERY_OORL parameter uint8; state_Engine input uint8; CD_VBATTERY_EXTREMELY_HIGH parameter uint8; CD_VBATTERY_DEBOUNCE_EXTREMELY_HIGH parameter uint16; HIGH parameter uint8; OORL parameter uint8; LOW parameter uint8; NORMAL parameter uint8; EXTREMELY_LOW parameter uint8; EXTREMELY_HIGH parameter uint8; ENGINE_CRANKING parameter uint8; NON_FUNCTIONAL parameter uint8; CD_VOLTAGE_HYSTERESIS parameter fixdt(0,8,4); CD_ALTERNATOR_FUNCTION parameter uint8; CD_ALTERNATOR_HIGH parameter uint8; CD_ALTERNATOR_LOW parameter uint8; state_Alternator output uint8; CD_ALTERNATOR_ALARM_ENGINE_SPEED parameter uint16; flt_EngineSpeed input uint16; CD_ALTERNATOR_DEBOUNCE_HIGH parameter uint16; CD_ALTERNATOR_DEBOUNCE_LOW parameter uint16

== stateflow chart MX_Gtwy_Control/Inputs/Engine_Start/Engine_Start ==
  state START_SERVICE_TOOL
    en:
    state_EngineStart = ACTIVE;
  state OFF
    en:
    state_EngineStart = INACTIVE;
  state START_MACHINE_KEY
    en:
    state_EngineStart = ACTIVE;
  state START_MACHINE_DELAY
    en:
    debounceDelay = 0;
    du:
    debounceDelay++;
  state START_ACD
    en:state_EngineStart = ACTIVE;
  (default) -> OFF
  OFF -> START_SERVICE_TOOL [order 3] : [(can_ST_EngineStart == ACTIVE)...
      | && (state_ST_CAN03 == NORMAL)]
  START_SERVICE_TOOL -> OFF : [((can_ST_EngineStart != ACTIVE) || (state_ST_CAN03 != NORMAL))]
  START_MACHINE_DELAY -> OFF [order 1] : [(debounceDelay >= CD_ENGINE_START_DELAY_OFF_TIME)...
      | || ((flt_EngineSpeed < CD_ENGINE_START_DELAY_RPM_NO_SIGNAL) && (debounceDelay >= CD_ENGINE_START_DELAY_DEBOUNCE_RPM_NO_SIGNAL))...
      | || (state_Engine == ENGINE_RUNNING)]
  OFF -> START_ACD [order 2] : [(can_ACD_EngineStart == ACTIVE)...
      | && (state_ACD_CAN03 == NORMAL)]
  START_ACD -> OFF : [(can_ACD_EngineStart != ACTIVE)...
      | || (state_ACD_CAN03 != NORMAL)]
  OFF -> START_MACHINE_KEY [order 1] : [(din_EngineStart == ACTIVE)...
      | && (state_MachineLock != ACTIVE)...
      | && (din_RemoteKey != ACTIVE)...
      | && (state_Engine != ENGINE_RUNNING)]
  START_MACHINE_KEY -> START_MACHINE_DELAY : [(din_EngineStart == INACTIVE)]
  START_MACHINE_DELAY -> START_MACHINE_KEY [order 2] : [(din_EngineStart == ACTIVE)]
  data: din_EngineStart input uint8; can_ST_EngineStart input uint8; can_ACD_EngineStart input uint8; state_EngineStart output uint8 =0; INACTIVE parameter uint8; ACTIVE parameter uint8; state_ST_CAN03 input uint8; state_ACD_CAN03 input uint8; NORMAL parameter uint8; state_MachineLock input uint8; din_RemoteKey input uint8; debounceDelay local uint16; CD_ENGINE_START_DELAY_OFF_TIME parameter uint16; state_Engine input uint8; flt_EngineSpeed input uint16; CD_ENGINE_START_DELAY_DEBOUNCE_RPM_NO_SIGNAL parameter uint16; CD_ENGINE_START_DELAY_RPM_NO_SIGNAL parameter uint16; ENGINE_RUNNING parameter uint8

== stateflow chart MX_Gtwy_Control/Inputs/Throttle/FastTravelThrottleLimit/Throttle_Limiter ==
  state Non_Functional
    en:
    out_Throttle = in_Throttle;
    du:
    out_Throttle = in_Throttle;
  state In_Neutral
    In_Neutral -> (junction 620) [order 1]
    (junction 620) -> (junction 621) [order 1] : [in_Throttle > CD_FAST_TRAVEL_THROTTLE_LIMIT]
    (junction 620) -> (junction 622) [order 2] : {
        | out_Throttle = in_Throttle;
        | }
    (junction 621) -> (junction 623) : {
        | out_Throttle = CD_FAST_TRAVEL_THROTTLE_LIMIT;
        | }
    (junction 623) -> (junction 622)
    (junction 622) -> (junction 624)
  state Debounce
    en:
    out_Throttle = in_Throttle;
    du:
    out_Throttle = in_Throttle;
  state Traveling
    en:
    out_Throttle = in_Throttle;
    du:
    out_Throttle = in_Throttle;
  (default) -> Non_Functional
  Non_Functional -> In_Neutral : [CD_FAST_TRAVEL_FUNCTION != NON_FUNCTIONAL]
  In_Neutral -> Traveling [order 1] : [adin_TravelPressureSwitch >= 512]
  Debounce -> In_Neutral [order 2] : [after(CD_FAST_TRAVEL_THROTTLE_DELAY,tick)]
  Traveling -> Debounce : [adin_TravelPressureSwitch < 512]
  Debounce -> Traveling [order 1] : [adin_TravelPressureSwitch >= 512]
  data: out_Throttle output uint16; in_Throttle input uint16; CD_FAST_TRAVEL_FUNCTION parameter uint8; NON_FUNCTIONAL parameter uint8; adin_TravelPressureSwitch input uint16; CD_FAST_TRAVEL_THROTTLE_LIMIT parameter uint16; CD_FAST_TRAVEL_THROTTLE_DELAY parameter uint16

== stateflow chart MX_Gtwy_Control/Inputs/HCFilter1/Hyd1_Filter_State ==
  state NON_FUNCTIONAL
    en:state_HCFilter1 = NORMAL;
  state NOT_CONNECTED
    state NOT_CONNECTED
      en:state_HCFilter1 = NOT_CONNECTED;
    state Debounce
      en:debounceCount = 0;
      du:debounceCount++;
    (default) -> NOT_CONNECTED.Debounce
    NOT_CONNECTED.Debounce -> NOT_CONNECTED.NOT_CONNECTED : [(debounceCount >= CD_HYD1_FILTER_DEBOUNCE_NOT_CONNECTED)...
        | || (state_HCFilter1 == NOT_CONNECTED)]
  state NORMAL_NO_CRANK
    state NORMAL
      en:state_HCFilter1 = NORMAL;
    state Debounce
      en:debounceCount = 0;
      du:debounceCount++;
    (default) -> NORMAL_NO_CRANK.Debounce
    NORMAL_NO_CRANK.Debounce -> NORMAL : [(debounceCount >= CD_HYD1_FILTER_DEBOUNCE_NORMAL)...
        | || (state_HCFilter1 == NORMAL)]
  note: Once we crank we do not want to be able to detecte NOT_CONNECTED again
      | because the system becomes pressurised and with could oil could trip a falase
      | not connected alarm.
  state NORMAL_AFTER_CRANK
    en:state_HCFilter1 = NORMAL;
  state DEBOUNCE_COLD
    en:debounceCount = 0;
    du:debounceCount++;
    ex:FilterDelay = ACTIVE;
  state PLUGGED
    state PLUGGED
      en:state_HCFilter1 = PLUGGED;
    state DEBOUNCE
      en:debounceCount = 0;
      du:debounceCount++;
    (default) -> DEBOUNCE
    DEBOUNCE -> PLUGGED.PLUGGED : [(debounceCount >= CD_HYD1_FILTER_DEBOUNCE_PLUGGED)]
  (default) -> NON_FUNCTIONAL
  NON_FUNCTIONAL -> NORMAL_NO_CRANK : [(CD_HYD1_FILTER_FUNCTION != NON_FUNCTIONAL)]
  NORMAL_NO_CRANK -> NOT_CONNECTED [order 2] : [(din_HCFilter1 == ACTIVE)...
      | && (state_Engine == ENGINE_NOT_RUNNING)]
  NOT_CONNECTED -> NORMAL_NO_CRANK : [(din_HCFilter1 != ACTIVE)]
  NORMAL_NO_CRANK -> NORMAL_AFTER_CRANK [order 1] : [(state_Engine == ENGINE_CRANKING)...
      | || (state_Engine == ENGINE_RUNNING)]
  NORMAL_AFTER_CRANK -> DEBOUNCE_COLD [order 1] : [(CD_HOT_FUNCTION != SENDER)...
      | && (state_Engine == ENGINE_RUNNING)...
      | && (FilterDelay == INACTIVE)]
  DEBOUNCE_COLD -> NORMAL_AFTER_CRANK : [(debounceCount >= CD_HYD_FILTER_DELAY)]
  NORMAL_AFTER_CRANK -> PLUGGED [order 2] : [(din_HCFilter1 == ACTIVE)...
      | && (((CD_HOT_FUNCTION == SENDER) && (state_HOT != COLD)) || ((CD_HOT_FUNCTION != SENDER) && (FilterDelay == ACTIVE)))...
      | && (state_Engine == ENGINE_RUNNING)]
  PLUGGED -> NORMAL_AFTER_CRANK : [((din_HCFilter1 == INACTIVE) || (state_HOT == COLD))...
      | && (state_HCFilter1 == NORMAL)]
  data: din_HCFilter1 input uint8; state_Engine input uint8; state_HCFilter1 output uint8; debounceCount local uint16; CD_HYD1_FILTER_FUNCTION parameter uint8; CD_HYD1_FILTER_DEBOUNCE_NORMAL parameter uint16; CD_HYD1_FILTER_DEBOUNCE_PLUGGED parameter uint16; state_HOT input uint8; CD_HOT_FUNCTION parameter uint8; FilterDelay local uint8 =0; CD_HYD_FILTER_DELAY parameter uint16; ACTIVE parameter uint8; INACTIVE parameter uint8; ENGINE_RUNNING parameter uint8; COLD parameter uint8; PLUGGED parameter uint8; NON_FUNCTIONAL parameter uint8; NORMAL parameter uint8; SENDER parameter uint8; NOT_CONNECTED parameter uint8; ENGINE_NOT_RUNNING parameter uint8; CD_HYD1_FILTER_DEBOUNCE_NOT_CONNECTED parameter uint16; ENGINE_CRANKING parameter uint8

== stateflow chart MX_Gtwy_Control/Inputs/Fuel_Temperature/ErrorDetection ==
  state NON_FUNCTIONAL
    en:state_FuelTemp = NORMAL;
  state FUNCTIONAL
    state SHUTDOWN
      state SHUTDOWN
        en:state_FuelTemp = SHUTDOWN;
      state DEBOUNCE
        en:debounceCount = 0;
        du:debounceCount++;
        en:state_FuelTemp = PRE_SHUTDOWN;
      (default) -> FUNCTIONAL.SHUTDOWN.DEBOUNCE
      FUNCTIONAL.SHUTDOWN.DEBOUNCE -> FUNCTIONAL.SHUTDOWN.SHUTDOWN : [(debounceCount >= CD_FUEL_TEMP_DEBOUNCE_SHUTDOWN)]
    state EXTREMELY_HIGH
      state DEBOUNCE
        en:debounceCount = 0;
        du:debounceCount++;
      state EXTREMELY_HIGH
        en:state_FuelTemp = EXTREMELY_HIGH;
      (default) -> FUNCTIONAL.EXTREMELY_HIGH.DEBOUNCE
      FUNCTIONAL.EXTREMELY_HIGH.DEBOUNCE -> FUNCTIONAL.EXTREMELY_HIGH.EXTREMELY_HIGH : [(debounceCount >= CD_FUEL_TEMP_DEBOUNCE_EXTREMELY_HIGH)...
          | || (state_FuelTemp == EXTREMELY_HIGH)]
    state HIGH
      state HIGH
        en:state_FuelTemp = HIGH;
      state DEBOUNCE
        en:debounceCount = 0;
        du:debounceCount++;
      (default) -> FUNCTIONAL.HIGH.DEBOUNCE
      FUNCTIONAL.HIGH.DEBOUNCE -> FUNCTIONAL.HIGH.HIGH : [(debounceCount >= CD_FUEL_TEMP_DEBOUNCE_HIGH)...
          | || (state_FuelTemp == HIGH)]
    state NORMAL
      state DEBOUNCE_NORMAL
        en:debounceCount = 0;
        du:debounceCount++;
      state NORMAL
        en:state_FuelTemp = NORMAL;
      (default) -> DEBOUNCE_NORMAL
      DEBOUNCE_NORMAL -> FUNCTIONAL.NORMAL.NORMAL : [(debounceCount >= CD_FUEL_TEMP_DEBOUNCE_NORMAL)...
          | || (state_FuelTemp == NORMAL)]
    state HEAT_SOAK
      state WAIT_FOR_RUNNING
        en:
        debounceCount = 0;
      state ENGINE_RUNNING
        en:
        debounceCount = 0;
        du:
        debounceCount++;
      (default) -> WAIT_FOR_RUNNING
      WAIT_FOR_RUNNING -> ENGINE_RUNNING : [(state_Engine == ENGINE_RUNNING)]
    FUNCTIONAL.EXTREMELY_HIGH -> FUNCTIONAL.SHUTDOWN [order 2] : [(CD_SHUTDOWN_FUNCTION != NON_FUNCTIONAL)...
        | && (can_FuelTemp >= CD_FUEL_TEMP_SHUTDOWN)]
    FUNCTIONAL.SHUTDOWN -> FUNCTIONAL.EXTREMELY_HIGH : [(state_FuelTemp != SHUTDOWN)...
        | && ((can_FuelTemp < (CD_FUEL_TEMP_SHUTDOWN - CD_TEMPERATURE_HYSTERESIS)) || (state_Engine != ENGINE_RUNNING))]
    FUNCTIONAL.EXTREMELY_HIGH -> FUNCTIONAL.HIGH [order 1] : [(can_FuelTemp < (CD_FUEL_TEMP_EXTREMELY_HIGH - CD_TEMPERATURE_HYSTERESIS))...
        | || (state_Engine != ENGINE_RUNNING)]
    FUNCTIONAL.HIGH -> FUNCTIONAL.EXTREMELY_HIGH [order 2] : [(can_FuelTemp >= CD_FUEL_TEMP_EXTREMELY_HIGH)...
        | && (state_FuelTemp == HIGH)]
    FUNCTIONAL.HIGH -> FUNCTIONAL.NORMAL [order 1] : [(can_FuelTemp < (CD_FUEL_TEMP_HIGH - CD_TEMPERATURE_HYSTERESIS))...
        | || (state_Engine != ENGINE_RUNNING)]
    FUNCTIONAL.NORMAL -> FUNCTIONAL.HIGH [order 1] : [(can_FuelTemp >= CD_FUEL_TEMP_HIGH)...
        | && (state_Engine == ENGINE_RUNNING)]
    (default) -> FUNCTIONAL.NORMAL
    FUNCTIONAL.NORMAL -> HEAT_SOAK [order 2] : [(state_Engine == ENGINE_CRANKING)]
    HEAT_SOAK -> FUNCTIONAL.NORMAL : [(debounceCount >= CD_FUEL_TEMP_DEBOUNCE_HEAT_SOAK)...
        | || ((state_Engine != ENGINE_RUNNING) && (state_Engine != ENGINE_CRANKING))]
  (default) -> NON_FUNCTIONAL
  NON_FUNCTIONAL -> FUNCTIONAL : [(CD_FUEL_TEMP_FUNCTION != NON_FUNCTIONAL)...
      | && (state_System == SYS_NORMAL)...
      | && (state_CommECU != NO_COMMUNICATION)]
  FUNCTIONAL -> NON_FUNCTIONAL : [(state_CommECU == NO_COMMUNICATION)]
  data: can_FuelTemp input uint16; state_FuelTemp output uint8; CD_FUEL_TEMP_DEBOUNCE_NORMAL parameter uint16; CD_FUEL_TEMP_DEBOUNCE_HIGH parameter uint16; CD_FUEL_TEMP_DEBOUNCE_EXTREMELY_HIGH parameter uint16; CD_FUEL_TEMP_DEBOUNCE_SHUTDOWN parameter uint16; CD_FUEL_TEMP_HIGH parameter uint16; CD_FUEL_TEMP_EXTREMELY_HIGH parameter uint16; debounceCount local uint16 =0; CD_SHUTDOWN_FUNCTION parameter uint8; CD_TEMPERATURE_HYSTERESIS parameter uint16; EXTREMELY_HIGH parameter uint8; HIGH parameter uint8; NON_FUNCTIONAL parameter uint8; NORMAL parameter uint8; SHUTDOWN parameter uint8; state_System input uint8; SYS_NORMAL parameter uint8; state_Engine input uint8; ENGINE_RUNNING parameter uint8; state_CommECU input uint8; CD_FUEL_TEMP_FUNCTION parameter uint8; NO_COMMUNICATION parameter uint8; CD_FUEL_TEMP_SHUTDOWN parameter uint16; PRE_SHUTDOWN parameter uint8; CD_FUEL_TEMP_DEBOUNCE_HEAT_SOAK parameter uint16; ENGINE_CRANKING parameter uint8
